simulink model "ospfb_2x_64kc_8i_16o"  (R2021a)
settings: Solver=VariableStepAuto  StartTime=0.0  StopTime=2304  RelTol=1e-3
undefined symbols (not defined in the file or in its folder): pfb_coeff_gen_calc
libraries: xbsIndex_r4 x37193, casper_library_misc x226, casper_library_delays x184
(part 183 of 313)

== system ospfb_2x_64kc_8i_16o/fft2/fft_direct/butterfly2_2/twiddle/coeff_gen/cosin ==
  theta : Inport
  misci : Inport  Port=2
  Constant4 : xbsIndex_r4/Constant  const=0  gui_display_data_type=Fixed-point  arith_type=Unsigned  n_bits=1  bin_pt=0  preci_type=Single  exp_width=8  frac_width=24  explicit_period=on  period=1  equ=P=C  opselect=C  inp2=PCIN>>17  opr=+  inp1=P  carry=CIN  dbl_ovrd=off  block_type=constant
  Constant5 : xbsIndex_r4/Constant  const=0  gui_display_data_type=Fixed-point  arith_type=Unsigned  n_bits=1  bin_pt=0  preci_type=Single  exp_width=8  frac_width=24  explicit_period=on  period=1  equ=P=C  opselect=C  inp2=PCIN>>17  opr=+  inp1=P  carry=CIN  dbl_ovrd=off  block_type=constant
  Delay : xbsIndex_r4/Delay  rst=off  infoeditControl=Selection of Reset will increase slice count due to use of real FFs and instead of SRLs  en=off  latency=bram_latency  reg_retiming=on  dbl_ovrd=off  block_type=delay
  Delay10 : xbsIndex_r4/Delay  rst=off  infoeditControl=Selection of Reset will increase slice count due to use of real FFs and instead of SRLs  en=off  latency=bram_latency  reg_retiming=on  dbl_ovrd=off  block_type=delay
  Delay8 : xbsIndex_r4/Delay  rst=off  infoeditControl=Selection of Reset will increase slice count due to use of real FFs and instead of SRLs  en=off  latency=bram_latency  reg_retiming=on  dbl_ovrd=off  block_type=delay
  ROM : xbsIndex_r4/ROM  depth=2^(12)  initVector=[24296199670 24291481060 24286762451 24282305985 24277587375 24272868765 24268150156 24263431546 24258975080 24254256470 24249537861 24244819251 24240100641 24235644175 24230925566 24226206956 24221488346 24216769736 24212051127 24207594661 24202876051 24198157442 24193438832 24188720222 24184001613 24179545147 24174826537 24170107928 24165389318 24160670708 24155952098 24151233489 24146514879 24142058413 24137339804 24132621194 24127902585 24123183975 24118465365 24113746756 24109028146 24104309536 24099590927 24095134461 24090415851 24085697242 24080978632 24076260023 24071541413 24066822803 24062104194 24057385584 24052666975 24047948365 24043229755 24038511146 24033792536 24029073927 24024355317 24019636708 24014918098 24010461632 24005743023 24001024413 23996305804 23991587194 23986868585 23982149975 23977431366 23972712756 23967994146 23963275537 23958556927 23953838318 23949119708 23944138955 23939420345 23934701736 23929983126 23925264517 23920545907 23915827298 23911108688 23906390079 23901671469 23896952860 23892234250 23887515641 23882797031 23878078422 23873359813 23868641203 23863922594 23858941840 23854223231 23849504621 23844786012 23840067402 23835348793 23830630184 23825911574 23821192965 23816474355 23811493602 23806774992 23802056383 23797337774 23792619164 23787900555 23783181945 23778463336 23773482583 23768763973 23764045364 23759326754 23754608145 23749889536 23744908782 23740190173 23735471564 23730752954 23726034345 23721315736 23716334982 23711616373 23706897763 23702179154 23697460545 23692479791 23687761182 23683042573 23678323964 23673605354 23668624601 23663905992 23659187382 23654468773 23649750164 23644769410 23640050801 23635332192 23630613582 23625632829 23620914220 23616195611 23611477001 23606496248 23601777639 23597059030 23592078276 23587359667 23582641058 23577922449 23572941695 23568223086 23563504477 23558523724 23553805114 23549086505 23544367896 23539387143 23534668533 23529949924 23524969171 23520250562 23515531953 23510551199 23505832590 23501113981 23496133228 23491414619 23486696010 23481715256 23476996647 23472278038 23467297285 23462578676 23457860067 23452879313 23448160704 23443179951 23438461342 23433742733 23428761980 23424043370 23419324761 23414344008 23409625399 23404644646 23399926037 23395207428 23390226675 23385508066 23380527312 23375808703 23370827950 23366109341 23361390732 23356409979 23351691370 23346710617 23341992008 23337011255 23332292646 23327574037 23322593283 23317874674 23312893921 23308175312 23303194559 23298475950 23293495197 23288776588 23283795835 23279077226 23274096473 23269377864 23264397111 23259678502 23254697749 23249979140 23244998387 23240279778 23235299025 23230580416 23225599663 23220881054 23215900301 23211181692 23206200939 23201482330 23196501577 23191782968 23186802215 23181821462 23177102853 23172122100 23167403491 23162422738 23157704129 23152723377 23148004768 23143024015 23138043262 23133324653 23128343900 23123625291 23118644538 23113663785 23108945176 23103964423 23099245814 23094265062 23089284309 23084565700 23079584947 23074866338 23069885585 23064904832 23060186223 23055205471 23050224718 23045506109 23040525356 23035544603 23030825994 23025845241 23021126633 23016145880 23011165127 23006446518 23001465765 22996485012 22991766404 22986785651 22981804898 22976824145 22972105536 22967124784 22962144031 22957425422 22952444669 22947463916 22942745308 22937764555 22932783802 22927803049 22923084441 22918103688 22913122935 22908404326 22903423574 22898442821 22893462068 22888743459 22883762707 22878781954 22873801201 22869082592 22864101840 22859121087 22854140334 22849421725 22844440973 22839460220 22834479467 22829498715 22824780106 22819799353 22814818601 22809837848 22804857095 22800138486 22795157734 22790176981 22785196228 22780215476 22775496867 22770516114 22765535362 22760554609 22755573857 22750593104 22745874495 22740893743 22735912990 22730932237 22725951485 22720970732 22716252124 22711271371 22706290618 22701309866 ...(+43449 chars)]  distributed_mem=Block RAM  rst=off  init_reg=0  en=off  latency=bram_latency  gui_display_data_type=Fixed-point  arith_type=Unsigned  n_bits=n_bits*2  bin_pt=0  preci_type=Single  exp_width=8  frac_width=24  optimize=Speed  use_rpm=on  dbl_ovrd=off  simulation_type=fast_sim  block_type=sprom
  Terminator : Terminator
  Terminator1 : Terminator
  Terminator4 : Terminator
  add_convert0 : Subsystem
  add_convert1 : Subsystem
  assert : xbsIndex_r4/Assert  assert_type=on  type_source=Explicitly  gui_display_data_type=Fixed-point  arith_type=Unsigned  n_bits=12  bin_pt=0  preci_type=Single  exp_width=8  frac_width=24  assert_rate=off  rate_source=Explicitly  period=1  output_port=on  block_type=assert
  c_to_ri : casper_library_misc/c_to_ri  n_bits=n_bits  bin_pt=bin_pt
      settings: SourceProductName=CASPER DSP Blockset
  invert0 : Subsystem  mask{n_bits=n_bits, bin_pt=bin_pt, neg_latency=neg_latency, mux_latency=mux_latency}
      mask prompts: n_bits "bit width"; bin_pt "binary point"; neg_latency "negate latency"; mux_latency "mux latency"
  invert1 : Subsystem  mask{n_bits=n_bits, bin_pt=bin_pt, neg_latency=neg_latency, mux_latency=mux_latency}
      mask prompts: n_bits "bit width"; bin_pt "binary point"; neg_latency "negate latency"; mux_latency "mux latency"
  cos : Outport
  -sin : Outport  Port=2
  misco : Outport  Port=3
  wires:
  theta/1 -> assert/1
  assert/1 -> add_convert0/1(theta), add_convert1/1(theta)
  Constant4/1 -> add_convert0/2(misci)
  add_convert0/3(misco) -> Terminator/1
  misci/1 -> add_convert1/2(misci)
  add_convert1/3(misco) -> Delay/1
  add_convert0/2(add) -> ROM/1
  add_convert1/2(add) -> Terminator4/1
  ROM/1 -> c_to_ri/1
  add_convert1/1(negate) -> Delay8/1
  add_convert0/1(negate) -> Delay10/1
  Delay10/1 -> invert0/1(negate)
  Constant5/1 -> invert0/3(misci)
  c_to_ri/1 -> invert0/2(in)
  Delay8/1 -> invert1/1(negate)
  c_to_ri/2 -> invert1/2(in)
  invert0/2(misco) -> Terminator1/1
  Delay/1 -> invert1/3(misci)
  invert0/1(out) -> cos/1
  invert1/1(out) -> -sin/1
  invert1/2(misco) -> misco/1
